simulink library "Renault_Library"  (Simulink 8.2)
settings: Solver=ode3  StartTime=0.0  StopTime=10.0  FixedStep=0.020  RelTol=1e-3
undefined symbols (not defined in the file or in its folder): ABC, AssystemProposal, b, chkval, CoeffA_C, CoeffB_C, CoeffMean_C, ConfTime_C, DiagDelay_C, DividePercent, FailureConfActive_C, FallingEdgeDuration_C, HighLevelThresholdVoltage_C, HighLevelVoltage_C, HighLevelVoltageTunable_C, InitValue_C, InputVoltRangeMax_C, InputVoltRangeMin_C, LowActiveContact_C, LowLevelThresholdVoltage_C, LowLevelVoltage_C, MaxOperatingVoltage_C, MinOperatingVoltage_C, mV2V_conversion, OUT1, OutOfRangeHigh_C, OutOfRangeLow_C, PinSupplySaturationMax, PinSupplySaturationMin, Res1Value_C, Res2Value_C, Res3Value_C, RisingEdgeDuration_C, RSA_CANSignalFailMonitoring, SampleTime_C, service14Table, service19Table, service22Table, service24Table, service28Table, service2ETable, service2FTable, service31Table, service87Table, SizeXStateDID2FTable, SizeXTable14, SizeXTable19, SizeXTable22, SizeXTable24, SizeXTable28, SizeXTable2E, SizeXTable2F, SizeXTable31, SizeXTable87, SizeYStateDID2FTable, SizeYTable14, SizeYTable19, SizeYTable22, SizeYTable24, SizeYTable28 ... (+22)
undefined symbols (not defined in the file): BatteryVoltage_ActivationThreshold_C, FrameStatus_AbsentValue_C, FrameStatus_ConfAbsentValue_C, MemThreshold_C, NAMEOFTHEMODEL_SAMPLE_TIME_CST, NbAbs1_C, NbConfAbs1_C, NbConfPres1_C, NbPres1_C, NOMDUSIGNAL_FaultConfTime_C, NOMDUSIGNAL_FaultRehabTime_C, NOMDUSIGNAL_switchvalue_C, NOMDUSIGNAL_unavailablevalue_C, PeriodFrame1_C, ResetTime_C, WakeUpTime_C
libraries: Renault_Library x112, simulink x75
(part 32 of 32)

== stateflow chart Services ECU/Diag/RSA_DiagnosticManagement/DIAGNOSTIC_SANCTION_TOP/Service28/Check_Message_Service28 ==
  (default) -> (junction 2131)
  (junction 2127) -> (junction 2128) [order 1] : [SUBFUNCTION_SUPPORTED_IN_ACTIVE_SESSION]
  (junction 2132) -> (junction 2127) [order 1] : [SUBFUNCTION_SUPPORTED]
  (junction 2128) -> (junction 2129) [order 1] : [PARAMETER_VALID]
  (junction 2129) -> (junction 2130) [order 1] : [CONDITION_CORRECT]
  (junction 2131) -> (junction 2132) [order 1] : [LENGTH_MESSAGE_CORRECT]
  (junction 2127) -> (junction 2135) [order 2] : {
      | NEGATIVE_RES_CODE= 0x7E ;
      | }
  (junction 2128) -> (junction 2136) [order 2] : {
      | NEGATIVE_RES_CODE= 0x31 ;
      | }
  (junction 2129) -> (junction 2137) [order 2] : {
      | NEGATIVE_RES_CODE= 0x22 ;
      | }
  (junction 2130) -> (junction 2138)
  (junction 2131) -> (junction 2133) [order 2] : {
      | NEGATIVE_RES_CODE= 0x13 ;
      | }
  (junction 2132) -> (junction 2134) [order 2] : {
      | NEGATIVE_RES_CODE= 0x12 ;
      | }
  (junction 2134) -> (junction 2133)
  (junction 2135) -> (junction 2134)
  (junction 2136) -> (junction 2135)
  (junction 2137) -> (junction 2136)
  (junction 2138) -> (junction 2137)
  (junction 2133) -> (junction 2139) : {
      |  SEND_RES_MESSAGE=!SEND_RES_MESSAGE;
      | }
  data: LENGTH_MESSAGE_CORRECT input boolean; CONDITION_CORRECT input boolean; NEGATIVE_RES_CODE output uint8; SUBFUNCTION_SUPPORTED_IN_ACTIVE_SESSION input boolean; PARAMETER_VALID input boolean; SUBFUNCTION_SUPPORTED input boolean; SEND_RES_MESSAGE output boolean

== stateflow chart Services ECU/Diag/RSA_DiagnosticManagement/DIAGNOSTIC_SANCTION_TOP/Service28/Parameter_Valid_Service28/Parameter_service28 ==
  (default) -> (junction 2167)
  (junction 2167) -> (junction 2170) : {
      |  i=0;...
      |  PARAMETER_VALID= false;...
      |  nodeIdentifierData=(DIAG_BUFFER_REQUEST[3]<<8) + DIAG_BUFFER_REQUEST[4];
      | }
  (junction 2168) -> (junction 2169) [order 1] : [nodeIdentifierData == service28Table[i][0]]
  (junction 2170) -> (junction 2168) [order 1] : [i< SizeXTable28]
  (junction 2168) -> (junction 2171) [order 2]
  (junction 2169) -> (junction 2172) : {
      |  PARAMETER_VALID= true;...
      |
      | }
  (junction 2171) -> (junction 2170) : {
      |  i ++;
      | }
  (junction 2170) -> (junction 2173) [order 2]
  (junction 2172) -> (junction 2171)
  data: nodeIdentifierData local uint16; DIAG_BUFFER_REQUEST input uint8 size=4095; PARAMETER_VALID output boolean; i local double; SizeYTable28 parameter double =5; SizeXTable28 parameter double; service28Table parameter double size=[SizeXTable28 SizeYTable28]

== stateflow chart Services ECU/Diag/RSA_DiagnosticManagement/DIAGNOSTIC_SANCTION_TOP/Service2E/Check_Message_Service2E ==
  (default) -> (junction 2192)
  (junction 2192) -> (junction 2193) [order 1] : [LENGTH_MESSAGE_CORRECT]
  (junction 2193) -> (junction 2194) [order 1] : [PARAMETER_VALID]
  (junction 2194) -> (junction 2195) [order 1] : [SECURITY_ACCESS_ACCEPTED]
  (junction 2195) -> (junction 2196) [order 1] : [CONDITION_CORRECT]
  (junction 2196) -> (junction 2197) [order 1] : [CORRECTLY_ALTERED_INTO_MEMORY]
  (junction 2192) -> (junction 2200) [order 2] : {
      | NEGATIVE_RES_CODE= 0x13 ;
      | }
  (junction 2193) -> (junction 2201) [order 2] : {
      | NEGATIVE_RES_CODE= 0x31 ;
      | }
  (junction 2194) -> (junction 2202) [order 2] : {
      | NEGATIVE_RES_CODE= 0x33 ;
      | }
  (junction 2195) -> (junction 2203) [order 2] : {
      | NEGATIVE_RES_CODE= 0x22 ;
      | }
  (junction 2196) -> (junction 2198) [order 2] : {
      | NEGATIVE_RES_CODE= 0x72 ;
      | }
  (junction 2197) -> (junction 2199)
  (junction 2199) -> (junction 2198)
  (junction 2201) -> (junction 2200)
  (junction 2198) -> (junction 2203)
  (junction 2202) -> (junction 2201)
  (junction 2203) -> (junction 2202)
  (junction 2200) -> (junction 2204) : {
      |  SEND_RES_MESSAGE=!SEND_RES_MESSAGE;
      | }
  data: LENGTH_MESSAGE_CORRECT input boolean; CONDITION_CORRECT input boolean; CORRECTLY_ALTERED_INTO_MEMORY input boolean; NEGATIVE_RES_CODE output uint8; SECURITY_ACCESS_ACCEPTED input boolean; PARAMETER_VALID input boolean; SEND_RES_MESSAGE output boolean

== stateflow chart Services ECU/Diag/RSA_DiagnosticManagement/DIAGNOSTIC_SANCTION_TOP/Service2E/Parameter_Valid_Service2E/Parameter_service2E ==
  state Session [function]
    (default) -> (junction 2226)
    (junction 2226) -> (junction 2227) [order 1] : [SESSION_ACTIVE==DEFAULT_SESSION]
        | {
        |  s=1;
        | }
    (junction 2226) -> (junction 2228) [order 2]
    (junction 2227) -> (junction 2229)
    (junction 2228) -> (junction 2229) [order 1] : [SESSION_ACTIVE==PROGRAMMING_SESSION]
        | {
        |  s=2;
        | }
    (junction 2228) -> (junction 2230) [order 2]
    (junction 2229) -> (junction 2231)
    (junction 2230) -> (junction 2231) [order 1] : [SESSION_ACTIVE==EXTENDED_SESSION]
        | {
        |  s=3;
        | }
    (junction 2230) -> (junction 2232) [order 2]
    (junction 2231) -> (junction 2233)
    (junction 2232) -> (junction 2233) [order 1] : [SESSION_ACTIVE==SAFETY_SYSTEM_SESSION]
        | {
        |  s=4;
        | }
    (junction 2232) -> (junction 2234) [order 2]
    (junction 2233) -> (junction 2235)
    (junction 2235) -> (junction 2234)
    (junction 2234) -> (junction 2236)
  (default) -> (junction 2266) : {
      |  j=0;...
      |  SECURITY_ACCESS_ACCEPTED=true;...
      |  PARAMETER_VALID= false;...
      |  number_bit_data=0;
      | }
  (junction 2264) -> (junction 2265) [order 1] : [DID == service2ETable[j][0] &&...
      |  service2ETable[j][s]==1  ]
  (junction 2266) -> (junction 2264) [order 1] : [j< SizeXTable2E ]
      | {
      | DID=(DIAG_BUFFER_REQUEST[1]<<8) + DIAG_BUFFER_REQUEST[2];...
      | Session();
      | }
  (junction 2264) -> (junction 2269) [order 2]
  (junction 2265) -> (junction 2267) : {
      |  number_bit_data+=service2ETable[j][6];
      | }
  (junction 2269) -> (junction 2266) : {
      |  j ++;
      | }
  (junction 2266) -> (junction 2275) [order 2]
  (junction 2267) -> (junction 2268) [order 1] : [uint8 ((number_bit_data+7)/8)==(REQ_DATA_NUMBER-3)]
  (junction 2267) -> (junction 2270) [order 2]
  (junction 2268) -> (junction 2271) : {
      |  PARAMETER_VALID= true;
      | }
  (junction 2270) -> (junction 2269)
  (junction 2273) -> (junction 2270)
  (junction 2271) -> (junction 2272) [order 1] : [service2ETable[j][5]==0]
  (junction 2271) -> (junction 2273) [order 2]
  (junction 2272) -> (junction 2274) : {
      |  SECURITY_ACCESS_ACCEPTED=false;
      | }
  (junction 2274) -> (junction 2273)
  data: DIAG_BUFFER_REQUEST input uint8 size=4095; REQ_DATA_NUMBER input uint8; PARAMETER_VALID output boolean; DID local uint16; j local uint32; service2ETable parameter double size=[SizeXTable2E SizeYTable2E]; SizeYTable2E parameter double =5; SizeXTable2E parameter double; SECURITY_ACCESS_ACCEPTED output boolean; SESSION_ACTIVE input Enum: TYP_SESSION_ACTIVE; s local double; number_bit_data local double

== stateflow chart Services ECU/Diag/RSA_DiagnosticManagement/DIAGNOSTIC_SANCTION_TOP/Service2F/Check_Message_Service2F ==
  (default) -> (junction 2301)
  (junction 2301) -> (junction 2300) [order 1] : [LENGTH_MESSAGE_CORRECT]
  (junction 2300) -> (junction 2302) [order 1] : [CONDITION_CORRECT]
  (junction 2302) -> (junction 2303) [order 1] : [PARAMETER_VALID]
  (junction 2303) -> (junction 2304) [order 1] : [SECURITY_ACCESS_ACCEPTED]
  (junction 2300) -> (junction 2305) [order 2] : {
      | NEGATIVE_RES_CODE= 0x22 ;
      | }
  (junction 2301) -> (junction 2306) [order 2] : {
      | NEGATIVE_RES_CODE= 0x13 ;
      | }
  (junction 2302) -> (junction 2307) [order 2] : {
      | NEGATIVE_RES_CODE= 0x31;
      | }
  (junction 2303) -> (junction 2308) [order 2] : {
      | NEGATIVE_RES_CODE= 0x33
      | }
  (junction 2304) -> (junction 2309)
  (junction 2307) -> (junction 2305)
  (junction 2305) -> (junction 2306)
  (junction 2308) -> (junction 2307)
  (junction 2309) -> (junction 2308)
  (junction 2306) -> (junction 2310) : {
      |  SEND_RES_MESSAGE=!SEND_RES_MESSAGE;
      | }
  data: LENGTH_MESSAGE_CORRECT input boolean; CONDITION_CORRECT input boolean; NEGATIVE_RES_CODE output uint8; SECURITY_ACCESS_ACCEPTED input boolean; PARAMETER_VALID input boolean; SEND_RES_MESSAGE output boolean

== stateflow chart Services ECU/Diag/RSA_DiagnosticManagement/DIAGNOSTIC_SANCTION_TOP/Service2F/Parameter_Valid_Service2F/Parameter_service2F ==
  state Session [function]
    (default) -> (junction 2329)
    (junction 2329) -> (junction 2330) [order 1] : [SESSION_ACTIVE==DEFAULT_SESSION]
        | {
        |  s=1;
        | }
    (junction 2329) -> (junction 2331) [order 2]
    (junction 2330) -> (junction 2332)
    (junction 2331) -> (junction 2332) [order 1] : [SESSION_ACTIVE==PROGRAMMING_SESSION]
        | {
        |  s=2;
        | }
    (junction 2331) -> (junction 2333) [order 2]
    (junction 2332) -> (junction 2334)
    (junction 2333) -> (junction 2334) [order 1] : [SESSION_ACTIVE==EXTENDED_SESSION]
        | {
        |  s=3;
        | }
    (junction 2333) -> (junction 2335) [order 2]
    (junction 2334) -> (junction 2336)
    (junction 2335) -> (junction 2336) [order 1] : [SESSION_ACTIVE==SAFETY_SYSTEM_SESSION]
        | {
        |  s=4;
        | }
    (junction 2335) -> (junction 2337) [order 2]
    (junction 2336) -> (junction 2338)
    (junction 2338) -> (junction 2337)
    (junction 2337) -> (junction 2339)
  (default) -> (junction 2369)
  (junction 2369) -> (junction 2375) : {
      |  i=0;
      |  j=0;...
      |  PARAMETER_VALID= false;...
      |  DID=(DIAG_BUFFER_REQUEST[1]<<8) + DIAG_BUFFER_REQUEST[2];
      | }
  (junction 2370) -> (junction 2371) [order 1] : [DID== stateDID2FTable[i][0] &&...
      |         DIAG_BUFFER_REQUEST[4]==stateDID2FTable[i][1]]
  (junction 2374) -> (junction 2370) [order 1] : [i<SizeXStateDID2FTable]
  (junction 2370) -> (junction 2378) [order 2]
  (junction 2371) -> (junction 2379) : {
      |  PARAMETER_VALID= true;...
      | }
  (junction 2372) -> (junction 2373) [order 1] : [DID== service2FTable[j][0] &&...
      |         service2FTable[j][s]==1]
  (junction 2373) -> (junction 2374) [order 1] : [DIAG_BUFFER_REQUEST[3]== 3 &&...
      |         service2FTable[j][9]==1 ]
  (junction 2375) -> (junction 2372) [order 1] : [j< SizeXTable2F]
      | {
      |  Session();
      | }
  (junction 2378) -> (junction 2374) : {
      | i++;
      | }
  (junction 2372) -> (junction 2382) [order 2]
  (junction 2373) -> (junction 2376) [order 2]
  (junction 2374) -> (junction 2386) [order 2]
  (junction 2382) -> (junction 2375) : {
      |  j ++;
      | }
  (junction 2375) -> (junction 2389) [order 2]
  (junction 2376) -> (junction 2377) [order 1] : [DIAG_BUFFER_REQUEST[3]== 2 &&...
      |         service2FTable[j][8]==1]
  (junction 2376) -> (junction 2380) [order 2]
  (junction 2377) -> (junction 2385) : {
      |  PARAMETER_VALID= true;...
      | }
  (junction 2379) -> (junction 2378)
  (junction 2380) -> (junction 2381) [order 1] : [DIAG_BUFFER_REQUEST[3]== 1 &&...
      |         service2FTable[j][7]==1]
  (junction 2380) -> (junction 2383) [order 2]
  (junction 2381) -> (junction 2384) : {
      |  PARAMETER_VALID= true;...
      | }
  (junction 2394) -> (junction 2382)
  (junction 2384) -> (junction 2383)
  (junction 2385) -> (junction 2384)
  (junction 2386) -> (junction 2385)
  (junction 2383) -> (junction 2387)
  (junction 2387) -> (junction 2388) [order 1] : [DIAG_BUFFER_REQUEST[3]== 0 &&...
      |         service2FTable[j][6]==1]
  (junction 2387) -> (junction 2390) [order 2]
  (junction 2388) -> (junction 2391) : {
      |  PARAMETER_VALID= true;...
      | }
  (junction 2391) -> (junction 2390)
  (junction 2390) -> (junction 2392)
  (junction 2392) -> (junction 2393) [order 1] : [service2FTable[j][5]==1]
  (junction 2392) -> (junction 2394) [order 2]
  (junction 2393) -> (junction 2395) : {
      |  SECURITY_ACCESS_ACCEPTED=true;
      | }
  (junction 2395) -> (junction 2394)
  data: SESSION_ACTIVE input Enum: TYP_SESSION_ACTIVE; SECURITY_ACCESS_ACCEPTED output double; DIAG_BUFFER_REQUEST input uint8 size=4095; PARAMETER_VALID output boolean; DID local uint16; j local uint32; service2FTable parameter double size=[SizeXTable2F SizeYTable2F]; s local double; SizeYTable2F parameter double; stateDID2FTable parameter double size=[SizeXStateDID2FTable SizeYStateDID2FTable]; SizeXTable2F parameter double; SizeXStateDID2FTable parameter double; SizeYStateDID2FTable parameter double; i local double

== stateflow chart Services ECU/Diag/RSA_DiagnosticManagement_v02.00/DIAGNOSTIC_SANCTION_TOP/Service31/Parameter_Valid_Service31/Parameter_service31 ==
  state Session [function]
    (default) -> (junction 2435)
    (junction 2435) -> (junction 2436) [order 1] : [SESSION_ACTIVE==DEFAULT_SESSION]
        | {
        |  s=1;
        | }
    (junction 2435) -> (junction 2437) [order 2]
    (junction 2436) -> (junction 2438)
    (junction 2437) -> (junction 2438) [order 1] : [SESSION_ACTIVE==PROGRAMMING_SESSION]
        | {
        |  s=2;
        | }
    (junction 2437) -> (junction 2439) [order 2]
    (junction 2438) -> (junction 2440)
    (junction 2439) -> (junction 2440) [order 1] : [SESSION_ACTIVE==EXTENDED_SESSION]
        | {
        |  s=3;
        | }
    (junction 2439) -> (junction 2441) [order 2]
    (junction 2440) -> (junction 2442)
    (junction 2441) -> (junction 2442) [order 1] : [SESSION_ACTIVE==SAFETY_SYSTEM_SESSION]
        | {
        |  s=4;
        | }
    (junction 2441) -> (junction 2443) [order 2]
    (junction 2442) -> (junction 2444)
    (junction 2444) -> (junction 2443)
    (junction 2443) -> (junction 2445)
  (default) -> (junction 2471)
  (junction 2471) -> (junction 2474) : {
      |  j=0;...
      |  PARAMETER_VALID= false;...
      |  SECURITY_ACCESS_ACCEPTED=false;...
      |  DID=(DIAG_BUFFER_REQUEST[2]<<8) + DIAG_BUFFER_REQUEST[3];
      | }
  (junction 2472) -> (junction 2473) [order 1] : [DID== service31Table[j][0] &&...
      |         service31Table[j][s]==1]
  (junction 2474) -> (junction 2472) [order 1] : [j< SizeXTable31 ]
      | {
      |  Session();
      | }
  (junction 2472) -> (junction 2477) [order 2]
  (junction 2473) -> (junction 2475) : {
      |  PARAMETER_VALID= true;...
      | }
  (junction 2477) -> (junction 2474) : {
      |  j ++;
      | }
  (junction 2474) -> (junction 2480) [order 2]
  (junction 2475) -> (junction 2476) [order 1] : [service31Table[j][5]==1]
  (junction 2475) -> (junction 2478) [order 2]
  (junction 2476) -> (junction 2479) : {
      |  SECURITY_ACCESS_ACCEPTED=true;
      | }
  (junction 2478) -> (junction 2477)
  (junction 2479) -> (junction 2478)
  data: SECURITY_ACCESS_ACCEPTED output double; DIAG_BUFFER_REQUEST input uint8 size=4095; PARAMETER_VALID output boolean; DID local uint16; j local uint32; service31Table parameter double size=[SizeXTable31 SizeYTable31]; SizeXTable31 parameter double; SESSION_ACTIVE input Enum: TYP_SESSION_ACTIVE; s local double; SizeYTable31 parameter double

== stateflow chart Services ECU/Diag/RSA_DiagnosticManagement/DIAGNOSTIC_SANCTION_TOP/Service31/Parameter_Valid_Service31/Parameter_service31 ==
  state Session [function]
    (default) -> (junction 2497)
    (junction 2497) -> (junction 2498) [order 1] : [SESSION_ACTIVE==DEFAULT_SESSION]
        | {
        |  s=1;
        | }
    (junction 2497) -> (junction 2499) [order 2]
    (junction 2498) -> (junction 2500)
    (junction 2499) -> (junction 2500) [order 1] : [SESSION_ACTIVE==PROGRAMMING_SESSION]
        | {
        |  s=2;
        | }
    (junction 2499) -> (junction 2501) [order 2]
    (junction 2500) -> (junction 2502)
    (junction 2501) -> (junction 2502) [order 1] : [SESSION_ACTIVE==EXTENDED_SESSION]
        | {
        |  s=3;
        | }
    (junction 2501) -> (junction 2503) [order 2]
    (junction 2502) -> (junction 2504)
    (junction 2503) -> (junction 2504) [order 1] : [SESSION_ACTIVE==SAFETY_SYSTEM_SESSION]
        | {
        |  s=4;
        | }
    (junction 2503) -> (junction 2505) [order 2]
    (junction 2504) -> (junction 2506)
    (junction 2506) -> (junction 2505)
    (junction 2505) -> (junction 2507)
  (default) -> (junction 2533)
  (junction 2533) -> (junction 2536) : {
      |  j=0;...
      |  PARAMETER_VALID= false;...
      |  SECURITY_ACCESS_ACCEPTED=false;...
      |  DID=(DIAG_BUFFER_REQUEST[2]<<8) + DIAG_BUFFER_REQUEST[3];
      | }
  (junction 2534) -> (junction 2535) [order 1] : [DID== service31Table[j][0] &&...
      |         service31Table[j][s]==1]
  (junction 2536) -> (junction 2534) [order 1] : [j< SizeXTable31 ]
      | {
      |  Session();
      | }
  (junction 2534) -> (junction 2539) [order 2]
  (junction 2535) -> (junction 2537) : {
      |  PARAMETER_VALID= true;...
      | }
  (junction 2539) -> (junction 2536) : {
      |  j ++;
      | }
  (junction 2536) -> (junction 2542) [order 2]
  (junction 2537) -> (junction 2538) [order 1] : [service31Table[j][5]==1]
  (junction 2537) -> (junction 2540) [order 2]
  (junction 2538) -> (junction 2541) : {
      |  SECURITY_ACCESS_ACCEPTED=true;
      | }
  (junction 2540) -> (junction 2539)
  (junction 2541) -> (junction 2540)
  data: SECURITY_ACCESS_ACCEPTED output double; DIAG_BUFFER_REQUEST input uint8 size=4095; PARAMETER_VALID output boolean; DID local uint16; j local uint32; service31Table parameter double size=[SizeXTable31 SizeYTable31]; SizeXTable31 parameter double; SESSION_ACTIVE input Enum: TYP_SESSION_ACTIVE; s local double; SizeYTable31 parameter double

== stateflow chart Services ECU/Diag/RSA_DiagnosticManagement/DIAGNOSTIC_SANCTION_TOP/Service3D/Check_Message_Service3D ==
  (default) -> (junction 2565)
  (junction 2565) -> (junction 2566) [order 1] : [LENGTH_MESSAGE_CORRECT]
  (junction 2566) -> (junction 2567) [order 1] : [PARAMETER_VALID]
  (junction 2567) -> (junction 2568) [order 1] : [SECURITY_ACCESS_ACCEPTED]
  (junction 2568) -> (junction 2569) [order 1] : [CONDITION_CORRECT]
  (junction 2569) -> (junction 2570) [order 1] : [CORRECTLY_ALTERED_INTO_MEMORY]
  (junction 2565) -> (junction 2573) [order 2] : {
      | NEGATIVE_RES_CODE= 0x13 ;
      | }
  (junction 2566) -> (junction 2574) [order 2] : {
      | NEGATIVE_RES_CODE= 0x31 ;
      | }
  (junction 2567) -> (junction 2575) [order 2] : {
      | NEGATIVE_RES_CODE= 0x33 ;
      | }
  (junction 2568) -> (junction 2576) [order 2] : {
      | NEGATIVE_RES_CODE= 0x22 ;
      | }
  (junction 2569) -> (junction 2571) [order 2] : {
      | NEGATIVE_RES_CODE= 0x72 ;
      | }
  (junction 2570) -> (junction 2572)
  (junction 2572) -> (junction 2571)
  (junction 2574) -> (junction 2573)
  (junction 2571) -> (junction 2576)
  (junction 2575) -> (junction 2574)
  (junction 2576) -> (junction 2575)
  (junction 2573) -> (junction 2577) : {
      |  SEND_RES_MESSAGE=!SEND_RES_MESSAGE;
      | }
  data: LENGTH_MESSAGE_CORRECT input boolean; CONDITION_CORRECT input boolean; CORRECTLY_ALTERED_INTO_MEMORY input boolean; NEGATIVE_RES_CODE output uint8; SECURITY_ACCESS_ACCEPTED input boolean; PARAMETER_VALID input boolean; SEND_RES_MESSAGE output boolean

== stateflow chart Services ECU/Diag/RSA_DiagnosticManagement_v02.00/DIAGNOSTIC_SANCTION_TOP/Service3D/Check_Message_Service3D ==
  (default) -> (junction 2605)
  (junction 2605) -> (junction 2606) [order 1] : [LENGTH_MESSAGE_CORRECT]
  (junction 2606) -> (junction 2607) [order 1] : [PARAMETER_VALID]
  (junction 2607) -> (junction 2608) [order 1] : [SECURITY_ACCESS_ACCEPTED]
  (junction 2608) -> (junction 2609) [order 1] : [CONDITION_CORRECT]
  (junction 2609) -> (junction 2610) [order 1] : [CORRECTLY_ALTERED_INTO_MEMORY]
  (junction 2605) -> (junction 2613) [order 2] : {
      | NEGATIVE_RES_CODE= 0x13 ;
      | }
  (junction 2606) -> (junction 2614) [order 2] : {
      | NEGATIVE_RES_CODE= 0x31 ;
      | }
  (junction 2607) -> (junction 2615) [order 2] : {
      | NEGATIVE_RES_CODE= 0x33 ;
      | }
  (junction 2608) -> (junction 2616) [order 2] : {
      | NEGATIVE_RES_CODE= 0x22 ;
      | }
  (junction 2609) -> (junction 2611) [order 2] : {
      | NEGATIVE_RES_CODE= 0x72 ;
      | }
  (junction 2610) -> (junction 2612)
  (junction 2612) -> (junction 2611)
  (junction 2614) -> (junction 2613)
  (junction 2611) -> (junction 2616)
  (junction 2615) -> (junction 2614)
  (junction 2616) -> (junction 2615)
  (junction 2613) -> (junction 2617) : {
      |  SEND_RES_MESSAGE=!SEND_RES_MESSAGE;
      | }
  data: LENGTH_MESSAGE_CORRECT input boolean; CONDITION_CORRECT input boolean; CORRECTLY_ALTERED_INTO_MEMORY input boolean; NEGATIVE_RES_CODE output uint8; SECURITY_ACCESS_ACCEPTED input boolean; PARAMETER_VALID input boolean; SEND_RES_MESSAGE output boolean

== stateflow chart Services ECU/Diag/RSA_DiagnosticManagement/DIAGNOSTIC_SANCTION_TOP/Service3E/Check_Message_Service3E ==
  (default) -> (junction 2645)
  (junction 2643) -> (junction 2644) [order 1] : [SUBFUNCTION_SUPPORTED_IN_ACTIVE_SESSION]
  (junction 2646) -> (junction 2643) [order 1] : [SUBFUNCTION_SUPPORTED]
  (junction 2645) -> (junction 2646) [order 1] : [LENGTH_MESSAGE_CORRECT]
  (junction 2643) -> (junction 2649) [order 2] : {
      | NEGATIVE_RES_CODE= 0x7E ;...
      | }
  (junction 2644) -> (junction 2650)
  (junction 2645) -> (junction 2647) [order 2] : {
      | NEGATIVE_RES_CODE= 0x13 ;...
      | }
  (junction 2646) -> (junction 2648) [order 2] : {
      | NEGATIVE_RES_CODE= 0x12 ;...
      | }
  (junction 2648) -> (junction 2647)
  (junction 2649) -> (junction 2648)
  (junction 2650) -> (junction 2649)
  (junction 2647) -> (junction 2651) : {
      |  SEND_RES_MESSAGE=!SEND_RES_MESSAGE;
      | }
  data: LENGTH_MESSAGE_CORRECT input boolean; SUBFUNCTION_SUPPORTED_IN_ACTIVE_SESSION input boolean; SUBFUNCTION_SUPPORTED input boolean; NEGATIVE_RES_CODE output uint8; SEND_RES_MESSAGE output boolean

== stateflow chart Services ECU/Diag/RSA_DiagnosticManagement/DIAGNOSTIC_SANCTION_TOP/Service87/Check_Message_Service87 ==
  (default) -> (junction 2679)
  (junction 2674) -> (junction 2675) [order 1] : [SUBFUNCTION_SUPPORTED_IN_ACTIVE_SESSION]
  (junction 2675) -> (junction 2676) [order 1] : [PARAMETER_VALID]
  (junction 2676) -> (junction 2677) [order 1] : [CONDITION_CORRECT]
  (junction 2677) -> (junction 2678) [order 1] : [REQ_SEQUENCE_OK]
  (junction 2680) -> (junction 2674) [order 1] : [SUBFUNCTION_SUPPORTED]
  (junction 2679) -> (junction 2680) [order 1] : [LENGTH_MESSAGE_CORRECT]
  (junction 2674) -> (junction 2683) [order 2] : {
      | NEGATIVE_RES_CODE= 0x7E ;
      | }
  (junction 2675) -> (junction 2684) [order 2] : {
      | NEGATIVE_RES_CODE= 0x31
      | }
  (junction 2676) -> (junction 2685) [order 2] : {
      | NEGATIVE_RES_CODE= 0x22 ;
      | }
  (junction 2677) -> (junction 2686) [order 2] : {
      | NEGATIVE_RES_CODE= 0x24 ;
      | }
  (junction 2678) -> (junction 2687)
  (junction 2679) -> (junction 2681) [order 2] : {
      | NEGATIVE_RES_CODE= 0x13 ;
      | }
  (junction 2680) -> (junction 2682) [order 2] : {
      | NEGATIVE_RES_CODE= 0x12 ;
      | }
  (junction 2682) -> (junction 2681)
  (junction 2683) -> (junction 2682)
  (junction 2684) -> (junction 2683)
  (junction 2685) -> (junction 2684)
  (junction 2686) -> (junction 2685)
  (junction 2687) -> (junction 2686)
  (junction 2681) -> (junction 2688) : {
      |  SEND_RES_MESSAGE=!SEND_RES_MESSAGE;
      | }
  data: LENGTH_MESSAGE_CORRECT input boolean; CONDITION_CORRECT input boolean; REQ_SEQUENCE_OK input boolean; NEGATIVE_RES_CODE output uint8; SUBFUNCTION_SUPPORTED_IN_ACTIVE_SESSION input boolean; PARAMETER_VALID input double; SUBFUNCTION_SUPPORTED input boolean; SEND_RES_MESSAGE output boolean

== stateflow chart Services ECU/Diag/RSA_DiagnosticManagement/DIAGNOSTIC_SANCTION_TOP/Service87/Parameter_Valid_Service87/Parameter_service87 ==
  (default) -> (junction 2721) : {
      |  j=0;...
      |  PARAMETER_VALID= false;...
      |  DID=(DIAG_BUFFER_REQUEST[2]<<8) + DIAG_BUFFER_REQUEST[3];
      | }
  (junction 2719) -> (junction 2720) [order 1] : [DIAG_BUFFER_REQUEST[1]==0x02 &&...
      |    DIAG_BUFFER_REQUEST[1]== service87Table[j][0] &&...
      |         (DIAG_BUFFER_REQUEST[2]<<16+DIAG_BUFFER_REQUEST[3]<<8+DIAG_BUFFER_REQUEST[4])==service87Table[j][1] ]
  (junction 2721) -> (junction 2719) [order 1] : [j< SizeXTable87 ]
      | {
      |  //Session();
      | }
  (junction 2719) -> (junction 2722) [order 2]
  (junction 2720) -> (junction 2726) : {
      |  PARAMETER_VALID= true;...
      | }
  (junction 2724) -> (junction 2721) : {
      |  j ++;
      | }
  (junction 2722) -> (junction 2723) [order 1] : [DIAG_BUFFER_REQUEST[1]==0x01 &&...
      |    DIAG_BUFFER_REQUEST[1]== service87Table[j][0] &&...
      |         DIAG_BUFFER_REQUEST[2]==service87Table[j][1] ]
  (junction 2721) -> (junction 2727) [order 2]
  (junction 2722) -> (junction 2724) [order 2]
  (junction 2723) -> (junction 2725) : {
      |  PARAMETER_VALID= true;...
      | }
  (junction 2725) -> (junction 2724)
  (junction 2726) -> (junction 2725)
  (junction 2727) -> (junction 2728) [order 1] : [DIAG_BUFFER_REQUEST[1]==0x03]
  (junction 2727) -> (junction 2729) [order 2]
  (junction 2728) -> (junction 2730) : {
      |  PARAMETER_VALID= true;...
      | }
  (junction 2730) -> (junction 2729)
  (junction 2729) -> (junction 2731)
  data: DIAG_BUFFER_REQUEST input uint8 size=4095; PARAMETER_VALID output boolean; DID local uint16; j local uint32; service87Table parameter double size=[SizeXTable87 SizeYTable87]; SizeXTable87 parameter double; SizeYTable87 parameter double

== stateflow chart Services ECU/Diag/RSA_DiagnosticManagement/DIAGNOSTIC_SANCTION_TOP/SessionDiagnostic/Diagnostic_Session_Control ==
  state DefaultSession/
    entry:
    SESSION_ACTIVE=DEFAULT_SESSION;
  state AnyOtherSession/
    entry:
    ChangeOfSession();
  state ChangeOfSession [function]
    (default) -> (junction 2754)
    (junction 2754) -> (junction 2755) [order 1] : [Session==1]
        | {
        |  SESSION_ACTIVE=DEFAULT_SESSION;
        | }
    (junction 2754) -> (junction 2756) [order 2]
    (junction 2755) -> (junction 2757)
    (junction 2756) -> (junction 2757) [order 1] : [Session==2]
        | {
        |  SESSION_ACTIVE=PROGRAMMING_SESSION;
        | }
    (junction 2756) -> (junction 2758) [order 2]
    (junction 2757) -> (junction 2759)
    (junction 2758) -> (junction 2759) [order 1] : [Session==3]
        | {
        |  SESSION_ACTIVE=EXTENDED_SESSION;
        | }
    (junction 2758) -> (junction 2760) [order 2]
    (junction 2759) -> (junction 2761)
    (junction 2760) -> (junction 2761) [order 1] : [Session==4]
        | {
        |  SESSION_ACTIVE=SAFETY_SYSTEM_SESSION;
        | }
    (junction 2760) -> (junction 2762) [order 2]
    (junction 2761) -> (junction 2763)
    (junction 2763) -> (junction 2762)
    (junction 2762) -> (junction 2764)
  (default) -> DefaultSession
  AnyOtherSession -> DefaultSession [order 1] : [Session==1 ...
      | || after(500,tick)]
  DefaultSession -> AnyOtherSession : [hasChanged(Session) ...
      |  && Session~=1 && Session~=0]
  AnyOtherSession -> AnyOtherSession [order 2] : [hasChanged(EV_TESTER_PRESENT) ...
      |  || (hasChanged(Session) && Session~=1 && Session~=0)]
  data: SESSION_ACTIVE output Enum: TYP_SESSION_ACTIVE; EV_TESTER_PRESENT input boolean; Session input uint8

== stateflow chart Services ECU/Diag/RSA_DiagnosticManagement/DIAGNOSTIC_SANCTION_TOP/SessionDiagnostic/Request_Of_Session ==
  state SESSION
    (default) -> (junction 2790) : [hasChanged(DIAG_BUFFER_REQUEST) && DIAG_BUFFER_REQUEST[0]!=0]
    (junction 2790) -> (junction 2791) [order 1] : [DIAG_BUFFER_REQUEST[0]==16 && DIAG_BUFFER_REQUEST[1]==1 && DefaultSession==0]
        | {
        |  Session=1;
        | }
    (junction 2791) -> (junction 2792)
    (junction 2790) -> (junction 2792) [order 2]
    (junction 2792) -> (junction 2793) [order 1] : [DIAG_BUFFER_REQUEST[0]==16 && DIAG_BUFFER_REQUEST[1]==2 && ProgrammingSession==0]
        | {
        |  Session=2;
        | }
    (junction 2793) -> (junction 2794)
    (junction 2792) -> (junction 2794) [order 2]
    (junction 2794) -> (junction 2795) [order 1] : [ DIAG_BUFFER_REQUEST[0]==16 && DIAG_BUFFER_REQUEST[1]==3 && ExtendedDiagnosticSession==0 ]
        | {
        |  Session=3;
        | }
    (junction 2795) -> (junction 2796)
    (junction 2794) -> (junction 2796) [order 2]
    (junction 2796) -> (junction 2797) [order 1] : [DIAG_BUFFER_REQUEST[0]==16 && DIAG_BUFFER_REQUEST[1]==4 && SafetySystemDiagnosticSession==0]
        | {
        |  Session=4;
        | }
    (junction 2797) -> (junction 2798)
    (junction 2796) -> (junction 2798) [order 2]
    (junction 2798) -> (junction 2799)
  (default) -> SESSION
  SESSION -> SESSION : [hasChanged(DIAG_BUFFER_REQUEST) ]
      | {
      |  Session=0;
      | }
  data: DIAG_BUFFER_REQUEST input uint8 size=4095; Session output uint8; DefaultSession constant double; ProgrammingSession constant double; ExtendedDiagnosticSession constant double; SafetySystemDiagnosticSession constant double

== stateflow chart Services ECU/Diag/RSA_DiagnosticManagement/DIAGNOSTIC_SANCTION_TOP/SessionDiagnostic/Tempo_Tester_Present ==
  state TESTER_PRESENT/
  (default) -> TESTER_PRESENT
  TESTER_PRESENT -> TESTER_PRESENT : [hasChanged(DIAG_BUFFER_REQUEST)...
      | && DIAG_BUFFER_REQUEST[0]==62 ...
      | &&(DIAG_BUFFER_REQUEST[1]==0 || DIAG_BUFFER_REQUEST[2]==128)]
      | {
      |  EV_TESTER_PRESENT=!EV_TESTER_PRESENT;
      | }
  data: DIAG_BUFFER_REQUEST input uint8 size=4095; EV_TESTER_PRESENT output boolean

== stateflow chart Services ECU/Diag/RSA_DiagnosticManagement_v02.00/DIAGNOSTIC_SANCTION_TOP/Service3D/Parameter_Valid_Service3D/Parameter_service3D ==
  state Session [function]
    (default) -> (junction 2832)
    (junction 2832) -> (junction 2833) [order 1] : [SESSION_ACTIVE==DEFAULT_SESSION]
        | {
        |  s=1;
        | }
    (junction 2832) -> (junction 2834) [order 2]
    (junction 2833) -> (junction 2835)
    (junction 2834) -> (junction 2835) [order 1] : [SESSION_ACTIVE==PROGRAMMING_SESSION]
        | {
        |  s=2;
        | }
    (junction 2834) -> (junction 2836) [order 2]
    (junction 2835) -> (junction 2837)
    (junction 2836) -> (junction 2837) [order 1] : [SESSION_ACTIVE==EXTENDED_SESSION]
        | {
        |  s=3;
        | }
    (junction 2836) -> (junction 2838) [order 2]
    (junction 2837) -> (junction 2839)
    (junction 2838) -> (junction 2839) [order 1] : [SESSION_ACTIVE==SAFETY_SYSTEM_SESSION]
        | {
        |  s=4;
        | }
    (junction 2838) -> (junction 2840) [order 2]
    (junction 2839) -> (junction 2841)
    (junction 2841) -> (junction 2840)
    (junction 2840) -> (junction 2842)
  (default) -> (junction 2867) : {
      |  i=1;...
      |  SECURITY_ACCESS_ACCEPTED=false;...
      |  PARAMETER_VALID= false;...
      |  memorySize=0;
      | }
  (junction 2867) -> (junction 2866) [order 1] : [i<=(DIAG_BUFFER_REQUEST[1]&0x0F)]
  (junction 2866) -> (junction 2868) : {
      |  memorySize+=DIAG_BUFFER_REQUEST[REQ_DATA_NUMBER-i]<<((i-1)*8);
      | }
  (junction 2868) -> (junction 2867) : {
      |  i ++;
      | }
  (junction 2867) -> (junction 2869) [order 2]
  (junction 2869) -> (junction 2870) [order 1] : [memorySize!=0 && ...
      |  memorySize<4095]
  (junction 2869) -> (junction 2871) [order 2]
  (junction 2870) -> (junction 2872) : {
      |  PARAMETER_VALID=true;
      | }
  (junction 2872) -> (junction 2871)
  (junction 2871) -> (junction 2873)
  data: DIAG_BUFFER_REQUEST input uint8 size=4095; REQ_DATA_NUMBER input uint8; PARAMETER_VALID output boolean; i local uint32; SECURITY_ACCESS_ACCEPTED output boolean; SESSION_ACTIVE input Enum: TYP_SESSION_ACTIVE; s local double; memorySize local double

== stateflow chart Services ECU/Diag/RSA_DiagnosticManagement_v02.00/DIAGNOSTIC_SANCTION_TOP/Service3E/Check_Message_Service3E ==
  (default) -> (junction 2893)
  (junction 2891) -> (junction 2892) [order 1] : [SUBFUNCTION_SUPPORTED_IN_ACTIVE_SESSION]
  (junction 2894) -> (junction 2891) [order 1] : [SUBFUNCTION_SUPPORTED]
  (junction 2893) -> (junction 2894) [order 1] : [LENGTH_MESSAGE_CORRECT]
  (junction 2891) -> (junction 2897) [order 2] : {
      | NEGATIVE_RES_CODE= 0x7E ;...
      | }
  (junction 2892) -> (junction 2898)
  (junction 2893) -> (junction 2895) [order 2] : {
      | NEGATIVE_RES_CODE= 0x13 ;...
      | }
  (junction 2894) -> (junction 2896) [order 2] : {
      | NEGATIVE_RES_CODE= 0x12 ;...
      | }
  (junction 2896) -> (junction 2895)
  (junction 2897) -> (junction 2896)
  (junction 2898) -> (junction 2897)
  (junction 2895) -> (junction 2899) : {
      |  SEND_RES_MESSAGE=!SEND_RES_MESSAGE;
      | }
  data: LENGTH_MESSAGE_CORRECT input boolean; SUBFUNCTION_SUPPORTED_IN_ACTIVE_SESSION input boolean; SUBFUNCTION_SUPPORTED input boolean; NEGATIVE_RES_CODE output uint8; SEND_RES_MESSAGE output boolean

== stateflow chart Services ECU/Diag/RSA_DiagnosticManagement_v02.00/DIAGNOSTIC_SANCTION_TOP/Service87/Check_Message_Service87 ==
  (default) -> (junction 2927)
  (junction 2922) -> (junction 2923) [order 1] : [SUBFUNCTION_SUPPORTED_IN_ACTIVE_SESSION]
  (junction 2923) -> (junction 2924) [order 1] : [PARAMETER_VALID]
  (junction 2924) -> (junction 2925) [order 1] : [CONDITION_CORRECT]
  (junction 2925) -> (junction 2926) [order 1] : [REQ_SEQUENCE_OK]
  (junction 2928) -> (junction 2922) [order 1] : [SUBFUNCTION_SUPPORTED]
  (junction 2927) -> (junction 2928) [order 1] : [LENGTH_MESSAGE_CORRECT]
  (junction 2922) -> (junction 2931) [order 2] : {
      | NEGATIVE_RES_CODE= 0x7E ;
      | }
  (junction 2923) -> (junction 2932) [order 2] : {
      | NEGATIVE_RES_CODE= 0x31
      | }
  (junction 2924) -> (junction 2933) [order 2] : {
      | NEGATIVE_RES_CODE= 0x22 ;
      | }
  (junction 2925) -> (junction 2934) [order 2] : {
      | NEGATIVE_RES_CODE= 0x24 ;
      | }
  (junction 2926) -> (junction 2935)
  (junction 2927) -> (junction 2929) [order 2] : {
      | NEGATIVE_RES_CODE= 0x13 ;
      | }
  (junction 2928) -> (junction 2930) [order 2] : {
      | NEGATIVE_RES_CODE= 0x12 ;
      | }
  (junction 2930) -> (junction 2929)
  (junction 2931) -> (junction 2930)
  (junction 2932) -> (junction 2931)
  (junction 2933) -> (junction 2932)
  (junction 2934) -> (junction 2933)
  (junction 2935) -> (junction 2934)
  (junction 2929) -> (junction 2936) : {
      |  SEND_RES_MESSAGE=!SEND_RES_MESSAGE;
      | }
  data: LENGTH_MESSAGE_CORRECT input boolean; CONDITION_CORRECT input boolean; REQ_SEQUENCE_OK input boolean; NEGATIVE_RES_CODE output uint8; SUBFUNCTION_SUPPORTED_IN_ACTIVE_SESSION input boolean; PARAMETER_VALID input double; SUBFUNCTION_SUPPORTED input boolean; SEND_RES_MESSAGE output boolean

== stateflow chart Services ECU/Diag/RSA_DiagnosticManagement_v02.00/DIAGNOSTIC_SANCTION_TOP/Service87/Parameter_Valid_Service87/Parameter_service87 ==
  (default) -> (junction 2969) : {
      |  j=0;...
      |  PARAMETER_VALID= false;...
      |  DID=(DIAG_BUFFER_REQUEST[2]<<8) + DIAG_BUFFER_REQUEST[3];
      | }
  (junction 2967) -> (junction 2968) [order 1] : [DIAG_BUFFER_REQUEST[1]==0x02 &&...
      |    DIAG_BUFFER_REQUEST[1]== service87Table[j][0] &&...
      |         (DIAG_BUFFER_REQUEST[2]<<16+DIAG_BUFFER_REQUEST[3]<<8+DIAG_BUFFER_REQUEST[4])==service87Table[j][1] ]
  (junction 2969) -> (junction 2967) [order 1] : [j< SizeXTable87 ]
      | {
      |  //Session();
      | }
  (junction 2967) -> (junction 2970) [order 2]
  (junction 2968) -> (junction 2974) : {
      |  PARAMETER_VALID= true;...
      | }
  (junction 2972) -> (junction 2969) : {
      |  j ++;
      | }
  (junction 2970) -> (junction 2971) [order 1] : [DIAG_BUFFER_REQUEST[1]==0x01 &&...
      |    DIAG_BUFFER_REQUEST[1]== service87Table[j][0] &&...
      |         DIAG_BUFFER_REQUEST[2]==service87Table[j][1] ]
  (junction 2969) -> (junction 2975) [order 2]
  (junction 2970) -> (junction 2972) [order 2]
  (junction 2971) -> (junction 2973) : {
      |  PARAMETER_VALID= true;...
      | }
  (junction 2973) -> (junction 2972)
  (junction 2974) -> (junction 2973)
  (junction 2975) -> (junction 2976) [order 1] : [DIAG_BUFFER_REQUEST[1]==0x03]
  (junction 2975) -> (junction 2977) [order 2]
  (junction 2976) -> (junction 2978) : {
      |  PARAMETER_VALID= true;...
      | }
  (junction 2978) -> (junction 2977)
  (junction 2977) -> (junction 2979)
  data: DIAG_BUFFER_REQUEST input uint8 size=4095; PARAMETER_VALID output boolean; DID local uint16; j local uint32; service87Table parameter double size=[SizeXTable87 SizeYTable87]; SizeXTable87 parameter double; SizeYTable87 parameter double

== stateflow chart Services ECU/Diag/RSA_DiagnosticManagement_v02.00/DIAGNOSTIC_SANCTION_TOP/SessionDiagnostic/Diagnostic_Session_Control ==
  state DefaultSession/
    entry:
    SESSION_ACTIVE=DEFAULT_SESSION;
  state AnyOtherSession/
    entry:
    ChangeOfSession();
  state ChangeOfSession [function]
    (default) -> (junction 3002)
    (junction 3002) -> (junction 3003) [order 1] : [Session==1]
        | {
        |  SESSION_ACTIVE=DEFAULT_SESSION;
        | }
    (junction 3002) -> (junction 3004) [order 2]
    (junction 3003) -> (junction 3005)
    (junction 3004) -> (junction 3005) [order 1] : [Session==2]
        | {
        |  SESSION_ACTIVE=PROGRAMMING_SESSION;
        | }
    (junction 3004) -> (junction 3006) [order 2]
    (junction 3005) -> (junction 3007)
    (junction 3006) -> (junction 3007) [order 1] : [Session==3]
        | {
        |  SESSION_ACTIVE=EXTENDED_SESSION;
        | }
    (junction 3006) -> (junction 3008) [order 2]
    (junction 3007) -> (junction 3009)
    (junction 3008) -> (junction 3009) [order 1] : [Session==4]
        | {
        |  SESSION_ACTIVE=SAFETY_SYSTEM_SESSION;
        | }
    (junction 3008) -> (junction 3010) [order 2]
    (junction 3009) -> (junction 3011)
    (junction 3011) -> (junction 3010)
    (junction 3010) -> (junction 3012)
  (default) -> DefaultSession
  AnyOtherSession -> DefaultSession [order 1] : [Session==1 ...
      | || after(500,tick)]
  DefaultSession -> AnyOtherSession : [hasChanged(Session) ...
      |  && Session~=1 && Session~=0]
  AnyOtherSession -> AnyOtherSession [order 2] : [hasChanged(EV_TESTER_PRESENT) ...
      |  || (hasChanged(Session) && Session~=1 && Session~=0)]
  data: SESSION_ACTIVE output Enum: TYP_SESSION_ACTIVE; EV_TESTER_PRESENT input boolean; Session input uint8

== stateflow chart Services ECU/Diag/RSA_DiagnosticManagement_v02.00/DIAGNOSTIC_SANCTION_TOP/SessionDiagnostic/Request_Of_Session ==
  state SESSION
    (default) -> (junction 3038) : [hasChanged(DIAG_BUFFER_REQUEST) && DIAG_BUFFER_REQUEST[0]!=0]
    (junction 3038) -> (junction 3039) [order 1] : [DIAG_BUFFER_REQUEST[0]==16 && DIAG_BUFFER_REQUEST[1]==1 && DefaultSession==0]
        | {
        |  Session=1;
        | }
    (junction 3039) -> (junction 3040)
    (junction 3038) -> (junction 3040) [order 2]
    (junction 3040) -> (junction 3041) [order 1] : [DIAG_BUFFER_REQUEST[0]==16 && DIAG_BUFFER_REQUEST[1]==2 && ProgrammingSession==0]
        | {
        |  Session=2;
        | }
    (junction 3041) -> (junction 3042)
    (junction 3040) -> (junction 3042) [order 2]
    (junction 3042) -> (junction 3043) [order 1] : [ DIAG_BUFFER_REQUEST[0]==16 && DIAG_BUFFER_REQUEST[1]==3 && ExtendedDiagnosticSession==0 ]
        | {
        |  Session=3;
        | }
    (junction 3043) -> (junction 3044)
    (junction 3042) -> (junction 3044) [order 2]
    (junction 3044) -> (junction 3045) [order 1] : [DIAG_BUFFER_REQUEST[0]==16 && DIAG_BUFFER_REQUEST[1]==4 && SafetySystemDiagnosticSession==0]
        | {
        |  Session=4;
        | }
    (junction 3045) -> (junction 3046)
    (junction 3044) -> (junction 3046) [order 2]
    (junction 3046) -> (junction 3047)
  (default) -> SESSION
  SESSION -> SESSION : [hasChanged(DIAG_BUFFER_REQUEST) ]
      | {
      |  Session=0;
      | }
  data: DIAG_BUFFER_REQUEST input uint8 size=4095; Session output uint8; DefaultSession constant double; ProgrammingSession constant double; ExtendedDiagnosticSession constant double; SafetySystemDiagnosticSession constant double

== stateflow chart Services ECU/Diag/RSA_DiagnosticManagement_v02.00/DIAGNOSTIC_SANCTION_TOP/SessionDiagnostic/Tempo_Tester_Present ==
  state TESTER_PRESENT/
  (default) -> TESTER_PRESENT
  TESTER_PRESENT -> TESTER_PRESENT : [hasChanged(DIAG_BUFFER_REQUEST)...
      | && DIAG_BUFFER_REQUEST[0]==62 ...
      | &&(DIAG_BUFFER_REQUEST[1]==0 || DIAG_BUFFER_REQUEST[2]==128)]
      | {
      |  EV_TESTER_PRESENT=!EV_TESTER_PRESENT;
      | }
  data: DIAG_BUFFER_REQUEST input uint8 size=4095; EV_TESTER_PRESENT output boolean

== stateflow chart Services ECU/Diag/RSA_DiagnosticManagement_v02.00/DIAGNOSTIC_SEGMENTATION_TOP/Diagnostic_Segmentation ==
  state DIAG_SEGMENTATION
    state IDLE_STATE/
      entry :
      SEG_STATE = TYP_SEG_STATE.IDLE_STATE;...
      REQ_NUMBER=0;...
      RES_NUMBER=0;...
      REQ_DATA_NUMBER=0;...
      DIAG_BUFFER_REQUEST=0;
    state PROCESSING/
      entry :
      REQ_DATA_NUMBER=FRAME_LENGHT;...
      SEG_STATE = TYP_SEG_STATE.PROCESSING_IN_PROGRESS;
    state REQUEST_IN_PROGRESS/
      entry :
      SEG_STATE = TYP_SEG_STATE.RECEIVE_IN_PROGRESS;
      state WAITING_CONSECUTIVE_FRAME/
        entry :
        RES_FRAME_TYPE=3;...
        UpdateDiagResponse();...
        REQ_NUMBER=REQ_NUMBER+1;...
        REQ_BS=0;
      state ANALYSE_CONSECUTIVE_FRAME/
        entry :
        REQ_BS=REQ_BS+1;
      (default) -> WAITING_CONSECUTIVE_FRAME
      ANALYSE_CONSECUTIVE_FRAME -> WAITING_CONSECUTIVE_FRAME [order 2] : [(REQ_FRAME_NUMBER!=REQ_NUMBER) && ...
          |  REQ_FRAME_SN==(REQ_NUMBER & 0x000F) &&...
          |  RES_FRAME_BS!=0 && ...
          |  RES_FRAME_BS==REQ_BS]
      WAITING_CONSECUTIVE_FRAME -> (junction 3113) [order 1] : [hasChanged(DTOOL_TO_ECU_FRAME_RECEIVED)]
          | /UpdateDiagRequest();
      (junction 3113) -> REQUEST_IN_PROGRESS [order 3] : [REQ_FRAME_TYPE==1]
      (junction 3113) -> ANALYSE_CONSECUTIVE_FRAME [order 2] : [REQ_FRAME_TYPE==2]
      (junction 3114) -> ANALYSE_CONSECUTIVE_FRAME [order 2] : [REQ_FRAME_TYPE==2 &&...
          | (RES_FRAME_BS==0 || ...
          |  REQ_BS<RES_FRAME_BS)]
      ANALYSE_CONSECUTIVE_FRAME -> (junction 3114) [order 1] : [hasChanged(DTOOL_TO_ECU_FRAME_RECEIVED)]
          | /UpdateDiagRequest();
      (junction 3114) -> REQUEST_IN_PROGRESS [order 3] : [REQ_FRAME_TYPE==1]
    state RESPONSE_IN_PROGRESS/
      entry:
      SEG_STATE = TYP_SEG_STATE.TRANSMIT_IN_PROGRESS;
      state ANALYSE_FLOW_CONTROL/
        entry :
        RES_BS=0;
      state WAITING_FLOW_CONTROL/
        entry :
        RES_NUMBER=RES_NUMBER+1;...
        RES_BS=RES_BS+1;
      (junction 3123) -> ANALYSE_FLOW_CONTROL : [REQ_FRAME_TYPE==3]
      ANALYSE_FLOW_CONTROL -> WAITING_FLOW_CONTROL [order 1] : after(REQ_FRAME_STMIN,tick)...
          | [REQ_FRAME_FS==0]
          | {RES_FRAME_TYPE=2;
          | UpdateDiagResponse();
          | }
      WAITING_FLOW_CONTROL -> (junction 3123) [order 1] : [hasChanged(DTOOL_TO_ECU_FRAME_RECEIVED)]
      WAITING_FLOW_CONTROL -> WAITING_FLOW_CONTROL [order 3] : after(REQ_FRAME_STMIN,tick)
          | [RES_NUMBER<(RES_FRAME_NUMBER+1) && ...
          | (REQ_FRAME_BS==0 || ...
          |  RES_BS<REQ_FRAME_BS)]
          | {RES_FRAME_TYPE=2;...
          | UpdateDiagResponse();}
    (default) -> IDLE_STATE
    WAITING_FLOW_CONTROL -> IDLE_STATE [order 2] : [RES_NUMBER==(RES_FRAME_NUMBER+1)]
    ANALYSE_CONSECUTIVE_FRAME -> IDLE_STATE [order 4] : [REQ_FRAME_SN!=(REQ_NUMBER & 0x000F)]
    PROCESSING -> IDLE_STATE [order 2] : after(TIME_OUT_TM_CST,tick)
    (junction 3092) -> IDLE_STATE [order 1] : [RES_DATA_NUMBER<=7 &&...
        |  DIAG_BUFFER_RESPONSE[0]!=0]
        | {RES_FRAME_TYPE=0;...
        | UpdateDiagResponse();
        | }
    (junction 3092) -> IDLE_STATE [order 3] : [RES_DATA_NUMBER==1&&...
        |  DIAG_BUFFER_RESPONSE[0]==0]
        | {ECU_TO_DTOOL_BYTE_0=0;...
        |  ECU_TO_DTOOL_BYTE_1=0;...
        |  ECU_TO_DTOOL_BYTE_2=0;...
        |  ECU_TO_DTOOL_BYTE_3=0;...
        |  ECU_TO_DTOOL_BYTE_4=0;...
        |  ECU_TO_DTOOL_BYTE_5=0;...
        |  ECU_TO_DTOOL_BYTE_6=0;...
        |  ECU_TO_DTOOL_BYTE_7=0;
        | }
    ANALYSE_FLOW_CONTROL -> IDLE_STATE [order 2] : [REQ_FRAME_FS!=0]
    IDLE_STATE -> (junction 3090) : [hasChanged(DTOOL_TO_ECU_FRAME_RECEIVED)]
        | /UpdateDiagRequest();
    (junction 3090) -> REQUEST_IN_PROGRESS [order 2] : [REQ_FRAME_TYPE==1]
    (junction 3090) -> PROCESSING [order 1] : [REQ_FRAME_TYPE==0]
    ANALYSE_FLOW_CONTROL -> DIAG_SEGMENTATION [order 3] : after(TIME_OUT_TM_CST,tick)
    WAITING_CONSECUTIVE_FRAME -> DIAG_SEGMENTATION [order 2] : after(TIME_OUT_TM_CST,tick)
    (junction 3113) -> PROCESSING [order 1] : [REQ_FRAME_TYPE==0]
    PROCESSING -> (junction 3091) [order 1] : [hasChanged(RES_DATA_NUMBER)]
    (junction 3091) -> (junction 3092) : [RES_DATA_NUMBER!=0]
    (junction 3092) -> WAITING_FLOW_CONTROL [order 2] : [RES_DATA_NUMBER>7]
        | {RES_FRAME_NUMBER=(RES_DATA_NUMBER/7);...
        | RES_FRAME_TYPE=1;...
        | UpdateDiagResponse();
        | }
    ANALYSE_CONSECUTIVE_FRAME -> PROCESSING [order 3] : [REQ_FRAME_SN==(REQ_NUMBER & 0x0F)  && ...
        | REQ_FRAME_NUMBER==REQ_NUMBER]
    ANALYSE_CONSECUTIVE_FRAME -> DIAG_SEGMENTATION [order 5] : after(TIME_OUT_TM_CST,tick)
    WAITING_FLOW_CONTROL -> DIAG_SEGMENTATION [order 4] : after(TIME_OUT_TM_CST,tick)
    (junction 3114) -> PROCESSING [order 1] : [REQ_FRAME_TYPE==0]
  state UpdateDiagResponse [function]
    (default) -> (junction 3128)
    (junction 3128) -> (junction 3129) [order 1] : [RES_FRAME_TYPE==0]
        | {ECU_TO_DTOOL_BYTE_0=(RES_DATA_NUMBER & 0x000F);...
        |  ECU_TO_DTOOL_BYTE_1=DIAG_BUFFER_RESPONSE[0];...
        |  ECU_TO_DTOOL_BYTE_2=DIAG_BUFFER_RESPONSE[1];...
        |  ECU_TO_DTOOL_BYTE_3=DIAG_BUFFER_RESPONSE[2];...
        |  ECU_TO_DTOOL_BYTE_4=DIAG_BUFFER_RESPONSE[3];...
        |  ECU_TO_DTOOL_BYTE_5=DIAG_BUFFER_RESPONSE[4];...
        |  ECU_TO_DTOOL_BYTE_6=DIAG_BUFFER_RESPONSE[5];...
        |  ECU_TO_DTOOL_BYTE_7=DIAG_BUFFER_RESPONSE[6];
        | }
    (junction 3128) -> (junction 3130) [order 2]
    (junction 3129) -> (junction 3131)
    (junction 3130) -> (junction 3131) [order 1] : [RES_FRAME_TYPE==1]
        | {ECU_TO_DTOOL_BYTE_0=0x10+((RES_DATA_NUMBER & 0x0F00)>>8);...
        |  ECU_TO_DTOOL_BYTE_1=(RES_DATA_NUMBER & 0x00FF);...
        |  ECU_TO_DTOOL_BYTE_2=DIAG_BUFFER_RESPONSE[0];...
        |  ECU_TO_DTOOL_BYTE_3=DIAG_BUFFER_RESPONSE[1];...
        |  ECU_TO_DTOOL_BYTE_4=DIAG_BUFFER_RESPONSE[2];...
        |  ECU_TO_DTOOL_BYTE_5=DIAG_BUFFER_RESPONSE[3];...
        |  ECU_TO_DTOOL_BYTE_6=DIAG_BUFFER_RESPONSE[4];...
        |  ECU_TO_DTOOL_BYTE_7=DIAG_BUFFER_RESPONSE[5];
        | }
    (junction 3130) -> (junction 3132) [order 2]
    (junction 3131) -> (junction 3133)
    (junction 3132) -> (junction 3133) [order 1] : [RES_FRAME_TYPE==2]
        | {RES_FRAME_SN=RES_NUMBER;...
        |  ECU_TO_DTOOL_BYTE_0=0x20+(RES_FRAME_SN & 0x000F);...
        |  ECU_TO_DTOOL_BYTE_1=DIAG_BUFFER_RESPONSE[RES_FRAME_SN*7-1];...
        |  ECU_TO_DTOOL_BYTE_2=DIAG_BUFFER_RESPONSE[RES_FRAME_SN*7];...
        |  ECU_TO_DTOOL_BYTE_3=DIAG_BUFFER_RESPONSE[RES_FRAME_SN*7+1];...
        |  ECU_TO_DTOOL_BYTE_4=DIAG_BUFFER_RESPONSE[RES_FRAME_SN*7+2];...
        |  ECU_TO_DTOOL_BYTE_5=DIAG_BUFFER_RESPONSE[RES_FRAME_SN*7+3];...
        |  ECU_TO_DTOOL_BYTE_6=DIAG_BUFFER_RESPONSE[RES_FRAME_SN*7+4];...
        |  ECU_TO_DTOOL_BYTE_7=DIAG_BUFFER_RESPONSE[RES_FRAME_SN*7+5];
        | }
    (junction 3132) -> (junction 3134) [order 2]
    (junction 3133) -> (junction 3135)
    (junction 3134) -> (junction 3135) [order 1] : [RES_FRAME_TYPE==3]
        | {ECU_TO_DTOOL_BYTE_0=0x30+RES_FRAME_FS;...
        |  ECU_TO_DTOOL_BYTE_1=RES_FRAME_BS;...
        |  ECU_TO_DTOOL_BYTE_2=RES_FRAME_STMIN;...
        |  ECU_TO_DTOOL_BYTE_3=0x00;...
        |  ECU_TO_DTOOL_BYTE_4=0x00;...
        |  ECU_TO_DTOOL_BYTE_5=0x00;...
        |  ECU_TO_DTOOL_BYTE_6=0x00;...
        |  ECU_TO_DTOOL_BYTE_7=0x00;
        | }
    (junction 3135) -> (junction 3136) : {ECU_TO_DTOOL_SEND=ECU_TO_DTOOL_SEND+1;}
    (junction 3134) -> (junction 3136) [order 2]
  state UpdateDiagRequest [function]
    (default) -> (junction 3150)
    (junction 3150) -> (junction 3151) [order 1] : [REQ_FRAME_TYPE==0]
        | {DIAG_BUFFER_REQUEST[0]=DTOOL_TO_ECU_BYTE_1;...
        |  DIAG_BUFFER_REQUEST[1]=DTOOL_TO_ECU_BYTE_2;...
        |  DIAG_BUFFER_REQUEST[2]=DTOOL_TO_ECU_BYTE_3;...
        |  DIAG_BUFFER_REQUEST[3]=DTOOL_TO_ECU_BYTE_4;...
        |  DIAG_BUFFER_REQUEST[4]=DTOOL_TO_ECU_BYTE_5;...
        |  DIAG_BUFFER_REQUEST[5]=DTOOL_TO_ECU_BYTE_6;...
        |  DIAG_BUFFER_REQUEST[6]=DTOOL_TO_ECU_BYTE_7;...
        |  FRAME_LENGHT=DTOOL_TO_ECU_BYTE_0 & 0x0F;
        |  }
    (junction 3150) -> (junction 3152) [order 2]
    (junction 3151) -> (junction 3153)
    (junction 3152) -> (junction 3153) [order 1] : [REQ_FRAME_TYPE==1]
        | {DIAG_BUFFER_REQUEST[0]=DTOOL_TO_ECU_BYTE_2;...
        |  DIAG_BUFFER_REQUEST[1]=DTOOL_TO_ECU_BYTE_3;...
        |  DIAG_BUFFER_REQUEST[2]=DTOOL_TO_ECU_BYTE_4;...
        |  DIAG_BUFFER_REQUEST[3]=DTOOL_TO_ECU_BYTE_5;...
        |  DIAG_BUFFER_REQUEST[4]=DTOOL_TO_ECU_BYTE_6;...
        |  DIAG_BUFFER_REQUEST[5]=DTOOL_TO_ECU_BYTE_7;...
        |  FRAME_LENGHT=((DTOOL_TO_ECU_BYTE_0 & 0x0F)<<8)+DTOOL_TO_ECU_BYTE_1;...
        |  REQ_FRAME_NUMBER=FRAME_LENGHT/7;
        |  }
    (junction 3152) -> (junction 3154) [order 2]
    (junction 3153) -> (junction 3155)
    (junction 3154) -> (junction 3155) [order 1] : [REQ_FRAME_TYPE==2]
        | {DIAG_BUFFER_REQUEST[REQ_NUMBER*7-1]=DTOOL_TO_ECU_BYTE_1;...
        |  DIAG_BUFFER_REQUEST[REQ_NUMBER*7]=DTOOL_TO_ECU_BYTE_2;...
        |  DIAG_BUFFER_REQUEST[REQ_NUMBER*7+1]=DTOOL_TO_ECU_BYTE_3;...
        |  DIAG_BUFFER_REQUEST[REQ_NUMBER*7+2]=DTOOL_TO_ECU_BYTE_4;...
        |  DIAG_BUFFER_REQUEST[REQ_NUMBER*7+3]=DTOOL_TO_ECU_BYTE_5;...
        |  DIAG_BUFFER_REQUEST[REQ_NUMBER*7+4]=DTOOL_TO_ECU_BYTE_6;......
        |  DIAG_BUFFER_REQUEST[REQ_NUMBER*7+5]=DTOOL_TO_ECU_BYTE_7;...
        |  REQ_FRAME_SN=DTOOL_TO_ECU_BYTE_0 & 0x0F;
        |  }
    (junction 3154) -> (junction 3156) [order 2]
    (junction 3155) -> (junction 3157)
    (junction 3156) -> (junction 3157) [order 1] : [REQ_FRAME_TYPE==3]
        | {REQ_FRAME_FS=DTOOL_TO_ECU_BYTE_0 & 0x0F;...
        |  REQ_FRAME_BS=DTOOL_TO_ECU_BYTE_1;...
        |  REQ_FRAME_STMIN=DTOOL_TO_ECU_BYTE_2;
        | }
    (junction 3157) -> (junction 3158)
    (junction 3156) -> (junction 3158) [order 2]
  data: DIAG_BUFFER_REQUEST output uint8 size=4095; REQ_DATA_NUMBER output uint16; ECU_TO_DTOOL_SEND output uint8; RES_FRAME_TYPE local uint8; DTOOL_TO_ECU_FRAME_RECEIVED input double; REQ_FRAME_TYPE input uint8; DTOOL_TO_ECU_BYTE_0 input uint8; TIME_OUT_TM_CST constant uint16 =90; REQ_BS local uint16 =0; REQ_NUMBER local uint16; RES_NUMBER local uint16; REQ_FRAME_NUMBER local uint8; REQ_FRAME_SN local uint8; REQ_FRAME_FS local uint8; REQ_FRAME_BS local uint8; REQ_FRAME_STMIN local uint8; SEG_STATE output Enum: TYP_SEG_STATE; RES_FRAME_BS constant uint8 =1; RES_FRAME_NUMBER local uint16; RES_BS local uint8; RES_FRAME_SN local uint16; DTOOL_TO_ECU_BYTE_1 input double; DTOOL_TO_ECU_BYTE_2 input double; DTOOL_TO_ECU_BYTE_3 input double; DTOOL_TO_ECU_BYTE_4 input double; DTOOL_TO_ECU_BYTE_5 input double; DTOOL_TO_ECU_BYTE_6 input double; DTOOL_TO_ECU_BYTE_7 input double; ECU_TO_DTOOL_BYTE_0 output uint8; ECU_TO_DTOOL_BYTE_1 output uint8; ECU_TO_DTOOL_BYTE_2 output uint8; ECU_TO_DTOOL_BYTE_3 output uint8; ECU_TO_DTOOL_BYTE_4 output uint8; ECU_TO_DTOOL_BYTE_5 output uint8; ECU_TO_DTOOL_BYTE_6 output uint8; ECU_TO_DTOOL_BYTE_7 output uint8; DIAG_BUFFER_RESPONSE input uint8 size=4095; RES_DATA_NUMBER input uint16; RES_FRAME_FS constant uint8 =0; RES_FRAME_STMIN constant uint8 =0; FRAME_LENGHT local uint16
